simulink model "ofdm_txrx_alamouti"  (Simulink 6.3)
settings: Solver=ode45  StartTime=0.0  StopTime=45000  RelTol=1e-3
InitFcn:
  | ofdm_rx_alamouti_init
StartFcn:
  | ofdm_rx_alamouti_init
values from neighbouring files (symbols this model uses):
  CPLength = 16    [ofdm_tx_alamouti_init.m]
  CRCPolynomial = hex2dec('04c11db7')    [calcTxCRC.m]    (also in ofdm_tx_alamouti_init.m)
  hi = zeros(1,length(cci))    [ofdm_rx_alamouti_init.m]
  hr = zeros(1,length(ccr))    [ofdm_rx_alamouti_init.m]
  max_CP_length = 16    [ofdm_tx_alamouti_init.m]
  max_num_baseRateSymbols = 31    [ofdm_tx_alamouti_init.m]
  max_num_subcarriers = 64    [ofdm_tx_alamouti_init.m]
  max_num_trainingSymbols = 15    [ofdm_tx_alamouti_init.m]
  max_OFDM_symbols = 2047    [ofdm_tx_alamouti_init.m]
  modConstellation_qam16 = 0.75*[1 3 -1 -3]./3    [ofdm_tx_alamouti_init.m]
  modConstellation_qam256 = 0.9375*[3 1 5 7 11 9 13 15 -3 -1 -5 -7 -11 -9 -13 -15]./15    [ofdm_tx_alamouti_init.m]
  modConstellation_qam64 = 0.875*[3 1 5 7 -3 -1 -5 -7]./7    [ofdm_tx_alamouti_init.m]
  modConstellation_qpsk = [1 -1]./sqrt(2)    [ofdm_tx_alamouti_init.m]
  numBaseRateSymbols = 2    [ofdm_tx_alamouti_init.m]
  numFullRateSymbols = numFullRateSymbols + mod(numFullRateSymbols, 2)    [ofdm_tx_alamouti_init.m]
  numSubcarriers = 64    [ofdm_tx_alamouti_init.m]
  numTrainingSymbols = 4    [ofdm_tx_alamouti_init.m]
  pkt_crossCorr_thresh = 0.7    [ofdm_rx_alamouti_init.m]
  pkt_energy_thresh = 0    [ofdm_rx_alamouti_init.m]
  pkt_numPayloadBytes = 24+4+20+20+500    (= 568)    [ofdm_tx_alamouti_init.m]
  pktTiming_count_payloadStart = pktTiming_count_lsStart + pktTiming_ls_num*pktTiming_ls_length    [ofdm_rx_init_packetTimingControl.m]
  preamble = 6*[0 shortsyms_10 longsyms_2]    [ofdm_tx_alamouti_init.m]
  preamble_I = real(preamble)    [ofdm_tx_alamouti_init.m]
  preamble_Q = imag(preamble)    [ofdm_tx_alamouti_init.m]
  RAM_init_size = 4096    [ofdm_tx_alamouti_init.m]
  rx_longCorrCountLoad = 251-16    (= 235)    [ofdm_rx_alamouti_init.m]
  rx_longCorrThresh = 3000    [ofdm_rx_alamouti_init.m]
  RxDataScrambling_Seq = [40 198 78 63 82 173 102 245 48 111 172 115 147 230 216 93 72 65 62 2 205 242 122 90 128 83 105 97 73 10 5 252]    [ofdm_tx_alamouti_init.m]
  simOnly_numSamples = length(preamble)+( (numSubcarriers+CPLength)*(numTrainingSymbols + numBaseRateSymbols + numFullRateSymbols) )    [ofdm_tx_alamouti_init.m]
  train = [train train]    [ofdm_tx_alamouti_init.m]
  TxDataScrambling_Seq = [40 198 78 63 82 173 102 245 48 111 172 115 147 230 216 93 72 65 62 2 205 242 122 90 128 83 105 97 73 10 5 252]    [ofdm_tx_alamouti_init.m]
undefined symbols (not defined in the file or in its folder): CRC_table_gen, gcbh, interpFilter_4, xlBlockLoadCallback
libraries: xbsIndex_r4 x4373, dspmlti4 x4, dspsigops x4, commchan3 x2, simulink x2, commrflib2 x2, commsink2 x2, dspsrcs4 x1
(part 1 of 39)
settings Model: LastModifiedBy=murphpo

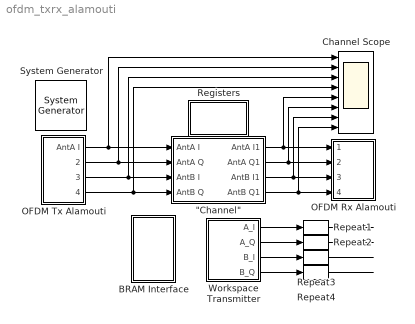
== system ofdm_txrx_alamouti ==
  " System Generator" : xbsIndex_r4/ System Generator  Tag=genX  xilinxfamily=Virtex2P  part=xc2vp70  speed=-6  package=ff1517  synthesis_tool=XST  directory=./opb_TxRx_Alamouti_2Rx_03  testbench=off  simulink_period=1  sysclk_period=20  incr_netlist=off  trim_vbits=Everywhere in SubSystem  dbl_ovrd=According to Block Masks  core_generation=According to Block Masks  run_coregen=off  deprecated_control=off  eval_field=0  block_type=sysgen
      settings: block_version=8.2.02
  "\"Channel\"" : Subsystem  mask{h=[1 0; 0 0], freqOffset=4e-4, awgnSNR=500, phNoise=-5000}
      mask prompts: h "2x2 Channel Matrix"; freqOffset "Freq Offset (phase inc [0,1])"; awgnSNR "SNR (dB)"; phNoise "Phase Noise"
      mask help: This block implements a simple static 2x2 channel. The one parameter sets the 2x2 channel matrix. h=[1 0;0 1] would be perfectly orthogonal channels. The norm of the matrix should be 1 to avoid overflow.
  BRAM Interface : Subsystem
  Channel Scope : Scope  NumInputPorts=8
      settings: TimeRange=10000  YMin=-1~-1~-1~-1~-3e-025~-3e-025~-3e-025~-3e-025  YMax=1~1~1~1~3e-025~3e-025~3e-025~3e-025  SaveName=ScopeData49
  OFDM Rx Alamouti : Subsystem
  OFDM Tx Alamouti : Subsystem
  Registers : Subsystem
  Repeat{1..4} (4 alike) : dspsigops/Repeat  N=4  ic=0  mode=Maintain input frame size
  Workspace Transmitter : Subsystem
  wires:
  OFDM Tx Alamouti/1(" AntA I") -> Channel Scope/1, "\"Channel\""/1(AntA I)
  OFDM Tx Alamouti/2(" AntA Q") -> "\"Channel\""/2(AntA Q), Channel Scope/2
  OFDM Tx Alamouti/3(" AntB I") -> "\"Channel\""/3(AntB I), Channel Scope/3
  OFDM Tx Alamouti/4(" AntB Q") -> "\"Channel\""/4(AntB Q), Channel Scope/4
  "\"Channel\""/1(AntA I1) -> Channel Scope/5, OFDM Rx Alamouti/1(AntA_I)
  "\"Channel\""/2(AntA Q1) -> Channel Scope/6, OFDM Rx Alamouti/2(AntA_Q)
  "\"Channel\""/3(AntB I1) -> Channel Scope/7, OFDM Rx Alamouti/3(AntB_I)
  "\"Channel\""/4(AntB Q1) -> Channel Scope/8, OFDM Rx Alamouti/4(AntB_Q)
  Workspace Transmitter/1(A_I) -> Repeat1/1
  Workspace Transmitter/2(A_Q) -> Repeat2/1
  Workspace Transmitter/3(B_I) -> Repeat3/1
  Workspace Transmitter/4(B_Q) -> Repeat4/1
  Repeat1/1 -> ?
  Repeat2/1 -> ?
  Repeat3/1 -> ?
  Repeat4/1 -> ?

== system ofdm_txrx_alamouti/"Channel" ==
  AntA I : Inport
  AntA Q : Inport  Port=2
  AntB I : Inport  Port=3
  AntB Q : Inport  Port=4
  AWGN Channel : commchan3/AWGN Channel  seed=rand  noiseMode="Signal to noise ratio  (SNR)"  EbNodB=10  EsNodB=10  SNRdB=awgnSNR  bitsPerSym=1  Ps=1  Tsym=1  variance=1
  AWGN Channel1 : commchan3/AWGN Channel  seed=rand  noiseMode="Signal to noise ratio  (SNR)"  EbNodB=10  EsNodB=10  SNRdB=awgnSNR  bitsPerSym=1  Ps=1  Tsym=1  variance=1
  Channel Scope : Scope  NumInputPorts=6
      settings: TimeRange=3000  YMin=-1~-1~-0.3~-0.3~-1~-1  YMax=0.75~0.75~0.2~0.2~1~1  SaveName=ScopeData52
  Complex to Real-Imag{1..5} (5 alike) : ComplexToRealImag
  FIR Decimation1 : dspmlti4/FIR Decimation  DialogController=dspDDGCreate  DialogControllerArgs=DataTag1  h=fir1(31, 1/4)  D=4  framing=Maintain input frame size  outputBufInitCond=0  roundingMode=Floor  overflowMode=off
  FIR Decimation2 : dspmlti4/FIR Decimation  DialogController=dspDDGCreate  DialogControllerArgs=DataTag2  h=fir1(31, 1/4)  D=4  framing=Maintain input frame size  outputBufInitCond=0  roundingMode=Floor  overflowMode=off
  FIR Decimation3 : dspmlti4/FIR Decimation  DialogController=dspDDGCreate  DialogControllerArgs=DataTag3  h=fir1(31, 1/4)  D=4  framing=Maintain input frame size  outputBufInitCond=0  roundingMode=Floor  overflowMode=off
  FIR Decimation4 : dspmlti4/FIR Decimation  DialogController=dspDDGCreate  DialogControllerArgs=DataTag4  h=fir1(31, 1/4)  D=4  framing=Maintain input frame size  outputBufInitCond=0  roundingMode=Floor  overflowMode=off
  Freq Offset : Subsystem
  Gain : Gain
  Gain1 : Gain
  Integer Delay : simulink/Discrete/Integer Delay  vinit=0.0  samptime=-1  NumDelays=1
  Integer Delay1 : simulink/Discrete/Integer Delay  vinit=0.0  samptime=-1  NumDelays=1
  "MIMO \"Channel\"" : Subsystem
  Phase Noise : commrflib2/Phase Noise  PhNs=phNoise  Fo=100  seed=rand
      settings: AttributesFormatString=\n
  Phase Noise1 : commrflib2/Phase Noise  PhNs=phNoise  Fo=100  seed=rand
      settings: AttributesFormatString=\n
  Real-Imag to Complex : RealImagToComplex
  Real-Imag to Complex1 : RealImagToComplex
  AntA I1 : Outport
  AntA Q1 : Outport  Port=2
  AntB I1 : Outport  Port=3
  AntB Q1 : Outport  Port=4
  wires:
  AntA I/1 -> Real-Imag to Complex/1
  AntA Q/1 -> Real-Imag to Complex/2
  AntB I/1 -> Real-Imag to Complex1/1
  AntB Q/1 -> Real-Imag to Complex1/2
  Complex to Real-Imag1/1 -> FIR Decimation3/1, AntA I1/1
  Complex to Real-Imag1/2 -> FIR Decimation4/1, AntA Q1/1
  "MIMO \"Channel\""/1(RxA) -> Freq Offset/1(In1), Complex to Real-Imag3/1
  Freq Offset/1(Out1) -> Gain/1, Complex to Real-Imag4/1
  "MIMO \"Channel\""/2(RxB) -> Freq Offset/2(In2)
  Gain/1 -> Integer Delay/1
  AWGN Channel/1 -> Complex to Real-Imag1/1
  Real-Imag to Complex/1 -> "MIMO \"Channel\""/1(TxA), Complex to Real-Imag2/1
  Real-Imag to Complex1/1 -> "MIMO \"Channel\""/2(TxB)
  Phase Noise/1 -> AWGN Channel/1
  FIR Decimation3/1 -> ?
  FIR Decimation4/1 -> ?
  Integer Delay/1 -> Phase Noise/1
  Complex to Real-Imag2/1 -> Channel Scope/1
  Complex to Real-Imag2/2 -> Channel Scope/2
  Complex to Real-Imag3/1 -> Channel Scope/3
  Complex to Real-Imag3/2 -> Channel Scope/4
  Complex to Real-Imag4/1 -> Channel Scope/5
  Complex to Real-Imag4/2 -> Channel Scope/6
  Complex to Real-Imag5/1 -> FIR Decimation1/1, AntB I1/1
  Complex to Real-Imag5/2 -> FIR Decimation2/1, AntB Q1/1
  Gain1/1 -> Integer Delay1/1
  AWGN Channel1/1 -> Complex to Real-Imag5/1
  Phase Noise1/1 -> AWGN Channel1/1
  FIR Decimation1/1 -> ?
  FIR Decimation2/1 -> ?
  Integer Delay1/1 -> Phase Noise1/1
  Freq Offset/2(Out2) -> Gain1/1

== system ofdm_txrx_alamouti/BRAM Interface ==
  " 19b1" : xbsIndex_r4/Constant  arith_type=Unsigned  const=0  n_bits=32-2-9-3  bin_pt=0  explicit_period=on  period=1  equ=P=C  opselect=C  inp2=PCIN>>17  opr=+  inp1=P  carry=CIN  dbl_ovrd=off  block_type=constant
      settings: block_version=8.2.02
  " 3b1" : xbsIndex_r4/Constant  arith_type=Unsigned  const=0  n_bits=3  bin_pt=0  explicit_period=on  period=1  equ=P=C  opselect=C  inp2=PCIN>>17  opr=+  inp1=P  carry=CIN  dbl_ovrd=off  block_type=constant
      settings: block_version=8.2.02
  19b1 : xbsIndex_r4/Constant  arith_type=Unsigned  const=0  n_bits=32-2-9-3  bin_pt=0  explicit_period=on  period=1  equ=P=C  opselect=C  inp2=PCIN>>17  opr=+  inp1=P  carry=CIN  dbl_ovrd=off  block_type=constant
      settings: block_version=8.2.02
  2LSB : xbsIndex_r4/Slice  nbits=2  boolean_output=off  mode=Lower Bit Location + Width  bit1=0  base1=MSB of Input  bit0=0  base0=LSB of Input  dbl_ovrd=off  block_type=slice
      settings: infoedit="Extracts a given range of bits from each input sample and presents it at the output.  The output type is ordinarily unsigned with binary point at zero, but can be Boolean when the slice is one bit wide.<P><P>Hardware notes: In hardware this block costs nothing."  block_version=VER_STRING_GOES_HERE
  2MSB : xbsIndex_r4/Slice  nbits=2  boolean_output=off  mode=Upper Bit Location + Width  bit1=0  base1=MSB of Input  bit0=0  base0=LSB of Input  dbl_ovrd=off  block_type=slice
      settings: infoedit="Extracts a given range of bits from each input sample and presents it at the output.  The output type is ordinarily unsigned with binary point at zero, but can be Boolean when the slice is one bit wide.<P><P>Hardware notes: In hardware this block costs nothing."  block_version=VER_STRING_GOES_HERE
  3b1 : xbsIndex_r4/Constant  arith_type=Unsigned  const=0  n_bits=3  bin_pt=0  explicit_period=on  period=1  equ=P=C  opselect=C  inp2=PCIN>>17  opr=+  inp1=P  carry=CIN  dbl_ovrd=off  block_type=constant
      settings: block_version=8.2.02
  BRAM_Addr : xbsIndex_r4/Gateway Out  hdl_port=on  timing_constraint=None  locs_specified=off  LOCs={}  block_type=gatewayout
      settings: infoedit="Gateway out block.  Converts Xilinx fixed point inputs into ouputs of type Simulink integer, double, or fixed point.<P><P>Hardware notes:  In hardware these blocks become top level output ports or are discarded, depending on how they are configured."  xl_area=[0, 0, 0, 0, 0, 0, 0]  block_version=VER_STRING_GOES_HERE
  BRAM_DataIn : xbsIndex_r4/Gateway In  arith_type=Unsigned  n_bits=64  bin_pt=0  quantization="Round  (unbiased: +/- Inf)"  overflow=Saturate  period=1  dbl_ovrd=off  timing_constraint=None  locs_specified=off  LOCs={}  block_type=gatewayin
      settings: infoedit="Gateway in block.  Converts inputs of type Simulink integer, double and fixed point to  Xilinx fixed point type.<P><P>Hardware notes:  In hardware these blocks become top level input ports."  block_version=VER_STRING_GOES_HERE
  BRAM_Dout : xbsIndex_r4/Gateway Out  hdl_port=on  timing_constraint=None  locs_specified=off  LOCs={}  block_type=gatewayout
      settings: infoedit="Gateway out block.  Converts Xilinx fixed point inputs into ouputs of type Simulink integer, double, or fixed point.<P><P>Hardware notes:  In hardware these blocks become top level output ports or are discarded, depending on how they are configured."  xl_area=[0, 0, 0, 0, 0, 0, 0]  block_version=VER_STRING_GOES_HERE
  BRAM_Reset : xbsIndex_r4/Gateway Out  hdl_port=on  timing_constraint=None  locs_specified=off  LOCs={}  block_type=gatewayout
      settings: infoedit="Gateway out block.  Converts Xilinx fixed point inputs into ouputs of type Simulink integer, double, or fixed point.<P><P>Hardware notes:  In hardware these blocks become top level output ports or are discarded, depending on how they are configured."  block_version=8.2.02
  BRAM_WEn : xbsIndex_r4/Gateway Out  hdl_port=on  timing_constraint=None  locs_specified=off  LOCs={}  block_type=gatewayout
      settings: infoedit="Gateway out block.  Converts Xilinx fixed point inputs into ouputs of type Simulink integer, double, or fixed point.<P><P>Hardware notes:  In hardware these blocks become top level output ports or are discarded, depending on how they are configured."  xl_area=[0, 0, 0, 0, 0, 0, 0]  block_version=VER_STRING_GOES_HERE
  Concat3 : xbsIndex_r4/Concat  num_inputs=4  dbl_ovrd=off  block_type=concat
      settings: block_version=8.2.02
  Concat4 : xbsIndex_r4/Concat  num_inputs=4  dbl_ovrd=off  block_type=concat
      settings: block_version=8.2.02
  Constant : xbsIndex_r4/Constant  arith_type=Unsigned  const=0  n_bits=1  bin_pt=0  explicit_period=on  period=1  equ=P=C  opselect=C  inp2=PCIN>>17  opr=+  inp1=P  carry=CIN  dbl_ovrd=off  block_type=constant
      settings: block_version=8.2.02
  Mux : xbsIndex_r4/Mux  inputs=2  en=off  latency=0  precision=Full  arith_type=Unsigned  n_bits=16  bin_pt=14  quantization=Truncate  overflow=Wrap  dbl_ovrd=off  block_type=mux
      settings: block_version=8.2.02
  PLB Pkt Buffer : Subsystem
  tag scope: BRAM_Tx_DIn=global, Debug_BRAM_Addr=global
  wires:
  PLB Pkt Buffer/1(Data) -> BRAM_DataIn/1
  [BRAM_Rx_DOut] -> BRAM_Dout/1
  [BRAM_Rx_WrEn] -> BRAM_WEn/1
  BRAM_DataIn/1 -> [BRAM_Tx_DIn]
  Constant/1 -> BRAM_Reset/1
  Mux/1 -> BRAM_Addr/1, [Debug_BRAM_Addr]
  [BRAM_Rx_AnyWrEn] -> Mux/1
  BRAM_Dout/1 -> PLB Pkt Buffer/1(BRAM_Dout)
  BRAM_WEn/1 -> PLB Pkt Buffer/2(WEn)
  BRAM_Addr/1 -> PLB Pkt Buffer/3(Addr)
  Concat4/1 -> Mux/2
  Concat3/1 -> Mux/3
  [BRAM_Rx_WrAddr] -> Concat3/3
  2MSB/1 -> Concat3/2
  19b1/1 -> Concat3/1
  " 3b1"/1 -> Concat4/4
  [BRAM_Tx_RdAddr] -> Concat4/3
  2LSB/1 -> Concat4/2
  " 19b1"/1 -> Concat4/1
  [regTxRx_PktBuffOffsets] -> 2LSB/1, 2MSB/1
  3b1/1 -> Concat3/4

== system ofdm_txrx_alamouti/OFDM Rx Alamouti ==
  AntA_I : Inport
  AntA_Q : Inport  Port=2
  AntB_I : Inport  Port=3
  AntB_Q : Inport  Port=4
  ADCs & Ant Selection : Subsystem
  ChipScope : xbsIndex_r4/ChipScope  num_trig_ports=5  current_port=4  show_trig_port=0  match_units=1  match_type=Basic with edges  data_is_trigger=off  num_data_ports=12  data_depth=8192  SRL16s=on  match_type_t0=2  match_type_t1=2  match_type_t2=2  match_type_t3=2  match_type_t4=2  match_type_t5=1  match_type_t6=1  match_type_t7=1  match_type_t8=1  match_type_t9=1  match_type_t10=1  match_type_t11=1  match_type_t12=1  match_type_t13=1  match_type_t14=1  match_type_t15=1  match_units_t0=1  match_units_t1=1  match_units_t2=1  match_units_t3=1  match_units_t4=1  match_units_t5=1  match_units_t6=1  match_units_t7=1  match_units_t8=1  match_units_t9=1  match_units_t10=1  match_units_t11=1  match_units_t12=1  match_units_t13=1  match_units_t14=1  match_units_t15=1  dbl_ovrd=0  block_type=chipscope
      settings: infoedit="Enables run-time debugging and verification of signals by inserting ChipScope Pro ICON and ILA cores.<br><br>  Restrictions: <br>Only one ChipScope core can be instantiated in a System Generator design.This block cannot be used at the same time as JTAG hardware co-simulation.A design or subsystem containing a ChipScope block must have at least one output port."  block_version=VER_STRING_GOES_HERE
  Coarse Freq Correction1 : Subsystem
  Constant : Constant  Value=0
  Counter1 : xbsIndex_r4/Counter  cnt_type=Free Running  cnt_to=Inf  operation=Up  start_count=0  cnt_by_val=1  arith_type=Unsigned  n_bits=2  bin_pt=0  load_pin=off  rst=off  en=off  explicit_period=on  period=1  dbl_ovrd=off  use_behavioral_HDL=off  use_rpm=on  block_type=counter
      settings: xl_area=[5 10 0 10 0 0 0]  block_version=VER_STRING_GOES_HERE
  Display : Display
  Display1 : Display
  Display2 : Display
  Divide : Product  Inputs=*/  OutScaling=2^-10
  Equalizer & Packetizer : Subsystem
  FFT & Chan Est : Subsystem
  Long Corr 4x : Subsystem
  Packet_Detection : Subsystem
  Packet_Timing_Control : Subsystem
  Rx Input : Scope  NumInputPorts=5
      settings: TimeRange=3500  YMin=-0.25~-0.25~0~0~0  YMax=0.25~0.25~1~12500~1  SaveName=ScopeData48
  Slice2 : xbsIndex_r4/Slice  nbits=1  boolean_output=off  mode=Upper Bit Location + Width  bit1=0  base1=MSB of Input  bit0=0  base0=LSB of Input  dbl_ovrd=off  block_type=slice
      settings: infoedit="Extracts a given range of bits from each input sample and presents it at the output.  The output type is ordinarily unsigned with binary point at zero, but can be Boolean when the slice is one bit wide.<P><P>Hardware notes: In hardware this block costs nothing."  block_version=VER_STRING_GOES_HERE
  Up Sample{,2,5,7,12} (5 alike) : xbsIndex_r4/Up Sample  sample_ratio=4  copy_samples=on  dbl_ovrd=off  block_type=usamp
      settings: infoedit="Up samples input data.  Inserted values  can be zeros or copies of the most recent input sample.<P><P>Hardware notes: No hardware is needed if inserted values are copies of the input sample; otherwise, a mux and single bit flip-flop are used."  block_version=VER_STRING_GOES_HERE
  Up Sample{1,3..4,6,9..11} (7 alike) : xbsIndex_r4/Up Sample  sample_ratio=2  copy_samples=on  dbl_ovrd=off  block_type=usamp
      settings: infoedit="Up samples input data.  Inserted values  can be zeros or copies of the most recent input sample.<P><P>Hardware notes: No hardware is needed if inserted values are copies of the input sample; otherwise, a mux and single bit flip-flop are used."  block_version=VER_STRING_GOES_HERE
  debug_chipscopeTrig : xbsIndex_r4/Gateway In  arith_type=Boolean  n_bits=14  bin_pt=13  quantization="Round  (unbiased: +/- Inf)"  overflow=Saturate  period=1  dbl_ovrd=off  timing_constraint=None  locs_specified=off  LOCs={}  block_type=gatewayin
      settings: infoedit="Gateway in block.  Converts inputs of type Simulink integer, double and fixed point to  Xilinx fixed point type.<P><P>Hardware notes:  In hardware these blocks become top level input ports."  block_version=VER_STRING_GOES_HERE
  done{1..2,4..5,13} (5 alike) : xbsIndex_r4/Gateway Out  hdl_port=off  timing_constraint=None  locs_specified=off  LOCs={}  block_type=gatewayout
      settings: infoedit="Gateway out block.  Converts Xilinx fixed point inputs into ouputs of type Simulink integer, double, or fixed point.<P><P>Hardware notes:  In hardware these blocks become top level output ports or are discarded, depending on how they are configured."  xl_area=[0 0 0 0 1 0 0]  block_version=VER_STRING_GOES_HERE
  tag scope: Payload=global
  wires:
  Packet_Detection/1(Pkt Det) -> done1/1, Packet_Timing_Control/1(Pkt Det)
  Packet_Timing_Control/1(Payload_Preamble) -> Equalizer & Packetizer/1(Payload), FFT & Chan Est/1(Payload), [Payload], done13/1
  Long Corr 4x/1(y) -> Packet_Timing_Control/2(Long Corr), done2/1
  done1/1 -> Rx Input/3
  done4/1 -> Rx Input/1
  done5/1 -> Rx Input/2
  done2/1 -> Rx Input/4
  Equalizer & Packetizer/1(Fine Freq) -> Coarse Freq Correction1/5(Fine Freq)
  Equalizer & Packetizer/2(done) -> Packet_Timing_Control/3(Done), Coarse Freq Correction1/6(PktDone)
  [Payload] -> Up Sample/1
  [AntA_ADC_I_Filt] -> Up Sample7/1
  [EQ_I] -> Up Sample3/1
  [EQ_Q] -> Up Sample4/1
  [Long_Corr] -> Up Sample2/1
  [FFT_I] -> Up Sample6/1
  Constant/1 -> debug_chipscopeTrig/1
  [ChanEst_I_EQ] -> Up Sample9/1
  Up Sample/1 -> ChipScope/6, ChipScope/1  "Payload"
  Up Sample2/1 -> ChipScope/13  "LongCorr"
  Up Sample3/1 -> ChipScope/11  "EQ_I"
  Up Sample4/1 -> ChipScope/12  "EQ_Q"
  Up Sample6/1 -> ChipScope/14  "FFT_I"
  Up Sample7/1 -> ChipScope/9  "AntA_ADC_I"
  debug_chipscopeTrig/1 -> ChipScope/2  "ExtTrig"
  Up Sample9/1 -> ChipScope/15  "ChanEstA I"
  Up Sample10/1 -> ChipScope/16  "PilotPhaseErr"
  FFT & Chan Est/1(Y_I) -> Equalizer & Packetizer/2(Y_I)
  FFT & Chan Est/2(Y_Q) -> Equalizer & Packetizer/3(Y_Q)
  FFT & Chan Est/3(H-I) -> Equalizer & Packetizer/4(H-I)
  FFT & Chan Est/4(H-Q) -> Equalizer & Packetizer/5(H-Q)
  FFT & Chan Est/5(SubCar Index) -> Equalizer & Packetizer/6(SubCar Index)
  FFT & Chan Est/6(Sym Index) -> Equalizer & Packetizer/7(Sym Index)
  FFT & Chan Est/7(Vout) -> Equalizer & Packetizer/8(Vin)
  Up Sample5/1 -> ChipScope/17  "CFO Phase Inc"
  [CFO_PhaseInc] -> Up Sample5/1
  Packet_Timing_Control/2(PktReset) -> ADCs & Ant Selection/5(PktDetReset), Coarse Freq Correction1/2(Reset)
  done13/1 -> Rx Input/5
  Coarse Freq Correction1/1(" AntA I") -> FFT & Chan Est/2(AntA I), Long Corr 4x/1(I In)
  Coarse Freq Correction1/2(" AntA Q") -> FFT & Chan Est/3(AntA Q), Long Corr 4x/2(Q In)
  [GoodPktInt] -> Up Sample1/1
  [BadPktInt] -> Up Sample11/1
  Up Sample1/1 -> ChipScope/7, ChipScope/3  "Good Pkt Int"
  Up Sample11/1 -> ChipScope/8, ChipScope/4  "Bad Pkt Int"
  [AntA_ADC_Q_Filt] -> Up Sample12/1
  Up Sample12/1 -> ChipScope/10  "AntA_ADC_Q"
  AntA_I/1 -> ADCs & Ant Selection/1("A I ")
  AntA_Q/1 -> ADCs & Ant Selection/2("A Q ")
  ADCs & Ant Selection/1(I) -> Packet_Detection/1(Ant I), done4/1, Coarse Freq Correction1/3(AntA I)
  ADCs & Ant Selection/2(Q) -> Packet_Detection/2(Ant Q), done5/1, Coarse Freq Correction1/4(AntA Q)
  AntB_I/1 -> ADCs & Ant Selection/3("B I ")
  AntB_Q/1 -> ADCs & Ant Selection/4("B Q ")
  [PilotPhaseErr] -> Up Sample10/1
  Counter1/1 -> Slice2/1
  Slice2/1 -> ChipScope/5  "SampClk"
  Divide/1 -> Display/1
  [BER_Errors] -> Divide/1, Display1/1
  [BER_TotalBits] -> Divide/2, Display2/1
  Packet_Timing_Control/3(PktDetected) -> Coarse Freq Correction1/1(PktDet)

== system ofdm_txrx_alamouti/OFDM Tx Alamouti ==
  Filter & DACs : Subsystem
  IFFT : Subsystem
  OutputBuffers : Subsystem
  OutputMuxes : Subsystem
  PreCFO : Subsystem
  Restarter : Subsystem
  Training_Data : Subsystem  Orientation=left
  Tx Scope : Scope  NumInputPorts=8
      settings: TimeRange=3000  YMin=-1~-1~-1~-0.0932617~0.0311279~-0.0801392~-1~-1  YMax=1~1~1~-0.0932617~0.0311279~-0.0801392~1~1  SaveName=ScopeData30
  TxControl : Subsystem
  TxStart : Subsystem
  done{1..8} (8 alike) : xbsIndex_r4/Gateway Out  hdl_port=off  timing_constraint=None  locs_specified=off  LOCs={}  block_type=gatewayout
      settings: infoedit="Gateway out block.  Converts Xilinx fixed point inputs into ouputs of type Simulink integer, double, or fixed point.<P><P>Hardware notes:  In hardware these blocks become top level output ports or are discarded, depending on how they are configured."  xl_area=[0 0 0 0 1 0 0]  block_version=VER_STRING_GOES_HERE
  " AntA I" : Outport
  " AntA Q" : Outport  Port=2
  " AntB I" : Outport  Port=3
  " AntB Q" : Outport  Port=4
  wires:
  Restarter/1(Start) -> TxControl/1(StartTx), Training_Data/5(start), done4/1
  Training_Data/1(tx_Q) -> IFFT/2(xn_Q), done8/1
  TxControl/6(Start IFFT) -> IFFT/3(Start), done2/1
  IFFT/3(xk_I) -> OutputBuffers/2(I), done6/1
  IFFT/4(xk_Q) -> OutputBuffers/3(Q)
  IFFT/1(xn_Index) -> Training_Data/7(xn_index)
  IFFT/5(xk_Index) -> OutputBuffers/4(Xk_index)
  OutputBuffers/1(AntA_I) -> OutputMuxes/2(AntA I), done3/1
  OutputBuffers/2(AntA_Q) -> OutputMuxes/3(AntA Q), done5/1
  TxControl/4(Training_Data) -> Training_Data/2(train_pay)
  TxControl/5(CPn_Data) -> Training_Data/1(CPn_Data)
  TxControl/3(BaseRate_En) -> Training_Data/3(BaseRate_En)
  OutputBuffers/5(Vout) -> OutputMuxes/6(vin)
  Training_Data/2(tx_I) -> IFFT/1(xn_I), done7/1
  OutputBuffers/6(Pkt Done) -> OutputMuxes/7(Pkt Done), Training_Data/6(packet_done), TxControl/2(PktDone), Restarter/2(Done), done1/1
  OutputBuffers/3(AntB_I) -> OutputMuxes/4(AntB I)
  OutputBuffers/4(AntB_Q) -> OutputMuxes/5(AntB Q)
  TxControl/1(Preamble Index) -> OutputMuxes/1(Pream Index)
  IFFT/2(Vout) -> OutputBuffers/1(IFFT Vout)
  TxControl/2(FFT En) -> Training_Data/4(FFT En)
  OutputMuxes/1(" AntA I") -> PreCFO/1(" AI")
  OutputMuxes/2(" AntA Q") -> PreCFO/2(" AQ")
  OutputMuxes/3(" AntB I") -> PreCFO/3(" BI")
  OutputMuxes/4(" AntB Q") -> PreCFO/4(" BQ")
  TxStart/1(Start) -> Restarter/1(StartTx)
  Restarter/2(2ndTx) -> OutputMuxes/8(2ndTx)
  done4/1 -> Tx Scope/1
  done1/1 -> Tx Scope/2
  done2/1 -> Tx Scope/3
  done3/1 -> Tx Scope/4
  done5/1 -> Tx Scope/5
  done6/1 -> Tx Scope/6
  done7/1 -> Tx Scope/7
  done8/1 -> Tx Scope/8
  PreCFO/1(AI) -> Filter & DACs/1(" AI")
  PreCFO/2(AQ) -> Filter & DACs/2(" AQ")
  PreCFO/3(BI) -> Filter & DACs/3(" BI")
  PreCFO/4(BQ) -> Filter & DACs/4(" BQ")
  Filter & DACs/1(AI) -> " AntA I"/1
  Filter & DACs/2(AQ) -> " AntA Q"/1
  Filter & DACs/3(BI) -> " AntB I"/1
  Filter & DACs/4(BQ) -> " AntB Q"/1

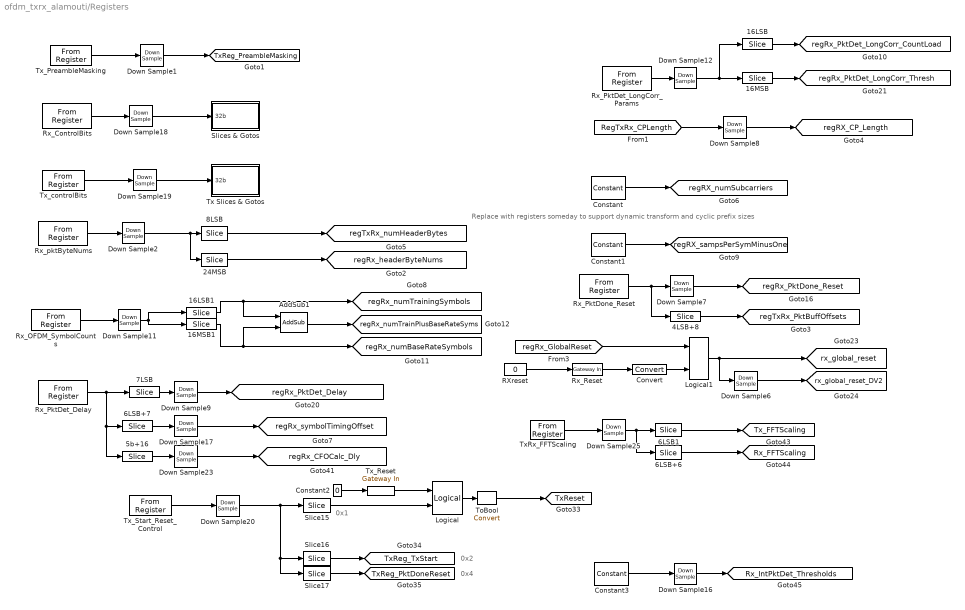
== system ofdm_txrx_alamouti/Registers ==
  note: Replace with registers someday
      | to support dynamic transform and
      | cyclic prefix sizes
  note: 0x2
  note: 0x1
  note: 0x4
  16LSB : xbsIndex_r4/Slice  nbits=16  boolean_output=off  mode=Lower Bit Location + Width  bit1=0  base1=MSB of Input  bit0=0  base0=LSB of Input  dbl_ovrd=off  block_type=slice
      settings: infoedit="Extracts a given range of bits from each input sample and presents it at the output.  The output type is ordinarily unsigned with binary point at zero, but can be Boolean when the slice is one bit wide.<P><P>Hardware notes: In hardware this block costs nothing."  block_version=VER_STRING_GOES_HERE
  16LSB1 : xbsIndex_r4/Slice  nbits=ceil(log2(max_num_trainingSymbols))  boolean_output=off  mode=Lower Bit Location + Width  bit1=0  base1=MSB of Input  bit0=0  base0=LSB of Input  dbl_ovrd=off  block_type=slice
      settings: infoedit="Extracts a given range of bits from each input sample and presents it at the output.  The output type is ordinarily unsigned with binary point at zero, but can be Boolean when the slice is one bit wide.<P><P>Hardware notes: In hardware this block costs nothing."  block_version=VER_STRING_GOES_HERE
  16MSB : xbsIndex_r4/Slice  nbits=16  boolean_output=off  mode=Lower Bit Location + Width  bit1=0  base1=MSB of Input  bit0=16  base0=LSB of Input  dbl_ovrd=off  block_type=slice
      settings: infoedit="Extracts a given range of bits from each input sample and presents it at the output.  The output type is ordinarily unsigned with binary point at zero, but can be Boolean when the slice is one bit wide.<P><P>Hardware notes: In hardware this block costs nothing."  block_version=VER_STRING_GOES_HERE
  16MSB1 : xbsIndex_r4/Slice  nbits=ceil(log2(max_num_baseRateSymbols))  boolean_output=off  mode=Lower Bit Location + Width  bit1=0  base1=MSB of Input  bit0=16  base0=LSB of Input  dbl_ovrd=off  block_type=slice
      settings: infoedit="Extracts a given range of bits from each input sample and presents it at the output.  The output type is ordinarily unsigned with binary point at zero, but can be Boolean when the slice is one bit wide.<P><P>Hardware notes: In hardware this block costs nothing."  block_version=VER_STRING_GOES_HERE
  24MSB : xbsIndex_r4/Slice  nbits=24  boolean_output=off  mode=Lower Bit Location + Width  bit1=0  base1=MSB of Input  bit0=8  base0=LSB of Input  dbl_ovrd=off  block_type=slice
      settings: infoedit="Extracts a given range of bits from each input sample and presents it at the output.  The output type is ordinarily unsigned with binary point at zero, but can be Boolean when the slice is one bit wide.<P><P>Hardware notes: In hardware this block costs nothing."  block_version=VER_STRING_GOES_HERE
  4LSB+8 : xbsIndex_r4/Slice  nbits=4  boolean_output=off  mode=Lower Bit Location + Width  bit1=0  base1=MSB of Input  bit0=8  base0=LSB of Input  dbl_ovrd=off  block_type=slice
      settings: infoedit="Extracts a given range of bits from each input sample and presents it at the output.  The output type is ordinarily unsigned with binary point at zero, but can be Boolean when the slice is one bit wide.<P><P>Hardware notes: In hardware this block costs nothing."  block_version=VER_STRING_GOES_HERE
  5b+16 : xbsIndex_r4/Slice  nbits=5  boolean_output=off  mode=Lower Bit Location + Width  bit1=0  base1=MSB of Input  bit0=16  base0=LSB of Input  dbl_ovrd=off  block_type=slice
      settings: infoedit="Extracts a given range of bits from each input sample and presents it at the output.  The output type is ordinarily unsigned with binary point at zero, but can be Boolean when the slice is one bit wide.<P><P>Hardware notes: In hardware this block costs nothing."  block_version=VER_STRING_GOES_HERE
  6LSB+6 : xbsIndex_r4/Slice  nbits=6  boolean_output=off  mode=Lower Bit Location + Width  bit1=0  base1=MSB of Input  bit0=6  base0=LSB of Input  dbl_ovrd=off  block_type=slice
      settings: infoedit="Extracts a given range of bits from each input sample and presents it at the output.  The output type is ordinarily unsigned with binary point at zero, but can be Boolean when the slice is one bit wide.<P><P>Hardware notes: In hardware this block costs nothing."  block_version=VER_STRING_GOES_HERE
  6LSB+7 : xbsIndex_r4/Slice  nbits=6  boolean_output=off  mode=Lower Bit Location + Width  bit1=0  base1=MSB of Input  bit0=7  base0=LSB of Input  dbl_ovrd=off  block_type=slice
      settings: infoedit="Extracts a given range of bits from each input sample and presents it at the output.  The output type is ordinarily unsigned with binary point at zero, but can be Boolean when the slice is one bit wide.<P><P>Hardware notes: In hardware this block costs nothing."  block_version=VER_STRING_GOES_HERE
  6LSB1 : xbsIndex_r4/Slice  nbits=6  boolean_output=off  mode=Lower Bit Location + Width  bit1=0  base1=MSB of Input  bit0=0  base0=LSB of Input  dbl_ovrd=off  block_type=slice
      settings: infoedit="Extracts a given range of bits from each input sample and presents it at the output.  The output type is ordinarily unsigned with binary point at zero, but can be Boolean when the slice is one bit wide.<P><P>Hardware notes: In hardware this block costs nothing."  block_version=VER_STRING_GOES_HERE
  7LSB : xbsIndex_r4/Slice  nbits=7  boolean_output=off  mode=Lower Bit Location + Width  bit1=0  base1=MSB of Input  bit0=0  base0=LSB of Input  dbl_ovrd=off  block_type=slice
      settings: infoedit="Extracts a given range of bits from each input sample and presents it at the output.  The output type is ordinarily unsigned with binary point at zero, but can be Boolean when the slice is one bit wide.<P><P>Hardware notes: In hardware this block costs nothing."  block_version=VER_STRING_GOES_HERE
  "8LSB " : xbsIndex_r4/Slice  nbits=8  boolean_output=off  mode=Lower Bit Location + Width  bit1=0  base1=MSB of Input  bit0=0  base0=LSB of Input  dbl_ovrd=off  block_type=slice
      settings: infoedit="Extracts a given range of bits from each input sample and presents it at the output.  The output type is ordinarily unsigned with binary point at zero, but can be Boolean when the slice is one bit wide.<P><P>Hardware notes: In hardware this block costs nothing."  block_version=VER_STRING_GOES_HERE
  AddSub1 : xbsIndex_r4/AddSub  mode=Addition  use_carryin=off  use_carryout=off  en=off  latency=0  precision=User Defined  arith_type=Unsigned  n_bits=1 + ceil(log2(max_num_trainingSymbols + max_num_baseRateSymbols))  bin_pt=0  quantization=Truncate  overflow=Wrap  dbl_ovrd=off  use_behavioral_HDL=on  pipelined=off  use_rpm=on  block_type=addsub
      settings: xl_area=[0, 0, 0, 0, 0, 0, 0]  block_version=VER_STRING_GOES_HERE
  Constant : xbsIndex_r4/Constant  arith_type=Unsigned  const=numSubcarriers  n_bits=ceil(log2(max_num_subcarriers)+1)  bin_pt=0  explicit_period=on  period=2  equ=P=C  opselect=C  inp2=PCIN>>17  opr=+  inp1=P  carry=CIN  dbl_ovrd=off  block_type=constant
      settings: block_version=VER_STRING_GOES_HERE
  Constant1 : xbsIndex_r4/Constant  arith_type=Unsigned  const=numSubcarriers+CPLength-1  n_bits=ceil(log2(max_num_subcarriers+CPLength)+1)  bin_pt=0  explicit_period=on  period=2  equ=P=C  opselect=C  inp2=PCIN>>17  opr=+  inp1=P  carry=CIN  dbl_ovrd=off  block_type=constant
      settings: block_version=VER_STRING_GOES_HERE
  Constant2 : Constant  Value=0
  Constant3 : xbsIndex_r4/Constant  arith_type=Unsigned  const=(2^8 * pkt_energy_thresh) + pkt_crossCorr_thresh  n_bits=32  bin_pt=0  explicit_period=on  period=1  equ=P=C  opselect=C  inp2=PCIN>>17  opr=+  inp1=P  carry=CIN  dbl_ovrd=off  block_type=constant
      settings: block_version=VER_STRING_GOES_HERE
  Convert : xbsIndex_r4/Convert  arith_type=Boolean  n_bits=8  bin_pt=6  quantization=Truncate  overflow=Wrap  latency=0  dbl_ovrd=off  pipeline=off  block_type=convert
      settings: xl_area=[0, 0, 0, 0, 0, 0, 0]  block_version=VER_STRING_GOES_HERE
  Down Sample{1,9,12,16,23} (5 alike) : xbsIndex_r4/Down Sample  sample_ratio=4  sample_phase="Last Value of Frame  (most efficient)"  en=off  latency=1  dbl_ovrd=off  block_type=dsamp
      settings: block_version=VER_STRING_GOES_HERE
  Down Sample{2,6..8,11,17..20,25} (10 alike) : xbsIndex_r4/Down Sample  sample_ratio=2  sample_phase="Last Value of Frame  (most efficient)"  en=off  latency=1  dbl_ovrd=off  block_type=dsamp
      settings: block_version=VER_STRING_GOES_HERE
  Logical : xbsIndex_r4/Logical  logical_function=OR  inputs=2  en=off  latency=0  precision=Full  arith_type=Unsigned  n_bits=16  bin_pt=0  align_bp=on  dbl_ovrd=off  block_type=logical
      settings: block_version=VER_STRING_GOES_HERE
  Logical1 : xbsIndex_r4/Logical  logical_function=OR  inputs=2  en=off  latency=0  precision=Full  arith_type=Unsigned  n_bits=8  bin_pt=2  align_bp=on  dbl_ovrd=off  block_type=logical
      settings: xl_area=[1 0 0 1 0 0 0]  block_version=VER_STRING_GOES_HERE
  RXreset : Constant  Value=0
  Rx_ControlBits : xbsIndex_r4/From Register  shared_memory_name='RxReg_ControlBits'  init=rx_controlBits  period=1  ownership=Locally owned and initialized  arith_type=Unsigned  n_bits=32  bin_pt=0  dbl_ovrd=off  block_type=fromreg
      settings: AttributesFormatString=<< %<shared_memory_name> >>  infoedit="Register block that reads data to a shared memory register.  Delay of one sample period."  block_version=VER_STRING_GOES_HERE
  Rx_OFDM_SymbolCounts : xbsIndex_r4/From Register  shared_memory_name='Rx_OFDM_SymbolCounts'  init=(2^16 * numBaseRateSymbols) + numTrainingSymbols  period=1  ownership=Locally owned and initialized  arith_type=Unsigned  n_bits=32  bin_pt=0  dbl_ovrd=off  block_type=fromreg
      settings: AttributesFormatString=<< %<shared_memory_name> >>  infoedit="Register block that reads data to a shared memory register.  Delay of one sample period."  block_version=VER_STRING_GOES_HERE
  Rx_PktDet_Delay : xbsIndex_r4/From Register  shared_memory_name='Rx_PktDet_Delay'  init=PktDet_Delay  period=1  ownership=Locally owned and initialized  arith_type=Unsigned  n_bits=32  bin_pt=0  dbl_ovrd=off  block_type=fromreg
      settings: AttributesFormatString=<< %<shared_memory_name> >>  infoedit="Register block that reads data to a shared memory register.  Delay of one sample period."  block_version=VER_STRING_GOES_HERE
  Rx_PktDet_LongCorr_Params : xbsIndex_r4/From Register  shared_memory_name='Rx_PktDet_LongCorr_Params'  init=rx_longCorrCountLoad + (2^16 * rx_longCorrThresh)  period=1  ownership=Locally owned and initialized  arith_type=Unsigned  n_bits=32  bin_pt=0  dbl_ovrd=off  block_type=fromreg
      settings: AttributesFormatString=<< %<shared_memory_name> >>  infoedit="Register block that reads data to a shared memory register.  Delay of one sample period."  block_version=VER_STRING_GOES_HERE
  Rx_PktDone_Reset : xbsIndex_r4/From Register  shared_memory_name='Rx_PktDone_Reset'  init=hex2dec('00') + (1 + 0)*2^8  period=1  ownership=Locally owned and initialized  arith_type=Unsigned  n_bits=32  bin_pt=0  dbl_ovrd=off  block_type=fromreg
      settings: AttributesFormatString=<< %<shared_memory_name> >>  infoedit="Register block that reads data to a shared memory register.  Delay of one sample period."  block_version=VER_STRING_GOES_HERE
  Rx_Reset : xbsIndex_r4/Gateway In  arith_type=Boolean  n_bits=6  bin_pt=0  quantization="Round  (unbiased: +/- Inf)"  overflow=Saturate  period=4  dbl_ovrd=off  timing_constraint=None  locs_specified=off  LOCs={}  block_type=gatewayin
      settings: infoedit="Gateway in block.  Converts inputs of type Simulink integer, double and fixed point to  Xilinx fixed point type.<P><P>Hardware notes:  In hardware these blocks become top level input ports."  xl_area=[0 0 0 0 1 0 0]  block_version=VER_STRING_GOES_HERE
  Rx_pktByteNums : xbsIndex_r4/From Register  shared_memory_name='Rx_pktByteNums'  init=pktByteNums  period=1  ownership=Locally owned and initialized  arith_type=Unsigned  n_bits=32  bin_pt=0  dbl_ovrd=off  block_type=fromreg
      settings: AttributesFormatString=<< %<shared_memory_name> >>  infoedit="Register block that reads data to a shared memory register.  Delay of one sample period."  block_version=VER_STRING_GOES_HERE
  Slice15 : xbsIndex_r4/Slice  nbits=1  boolean_output=off  mode=Lower Bit Location + Width  bit1=0  base1=MSB of Input  bit0=0  base0=LSB of Input  dbl_ovrd=off  block_type=slice
      settings: infoedit="Extracts a given range of bits from each input sample and presents it at the output.  The output type is ordinarily unsigned with binary point at zero, but can be Boolean when the slice is one bit wide.<P><P>Hardware notes: In hardware this block costs nothing."  block_version=VER_STRING_GOES_HERE
  Slice16 : xbsIndex_r4/Slice  nbits=1  boolean_output=on  mode=Lower Bit Location + Width  bit1=0  base1=MSB of Input  bit0=1  base0=LSB of Input  dbl_ovrd=off  block_type=slice
      settings: infoedit="Extracts a given range of bits from each input sample and presents it at the output.  The output type is ordinarily unsigned with binary point at zero, but can be Boolean when the slice is one bit wide.<P><P>Hardware notes: In hardware this block costs nothing."  block_version=VER_STRING_GOES_HERE
  Slice17 : xbsIndex_r4/Slice  nbits=1  boolean_output=on  mode=Lower Bit Location + Width  bit1=0  base1=MSB of Input  bit0=2  base0=LSB of Input  dbl_ovrd=off  block_type=slice
      settings: infoedit="Extracts a given range of bits from each input sample and presents it at the output.  The output type is ordinarily unsigned with binary point at zero, but can be Boolean when the slice is one bit wide.<P><P>Hardware notes: In hardware this block costs nothing."  block_version=VER_STRING_GOES_HERE
  Slices & Gotos : Subsystem
  ToBool : xbsIndex_r4/Convert  arith_type=Boolean  n_bits=16  bin_pt=14  quantization=Truncate  overflow=Wrap  latency=0  dbl_ovrd=off  pipeline=off  block_type=convert
      settings: block_version=VER_STRING_GOES_HERE
  Tx Slices & Gotos : Subsystem
  TxRx_FFTScaling : xbsIndex_r4/From Register  shared_memory_name='TxRx_FFT_Scaling'  init=TxRx_FFTScaling  period=1  ownership=Locally owned and initialized  arith_type=Unsigned  n_bits=12  bin_pt=0  dbl_ovrd=off  block_type=fromreg
      settings: AttributesFormatString=<< %<shared_memory_name> >>  infoedit="Register block that reads data to a shared memory register.  Delay of one sample period."  block_version=VER_STRING_GOES_HERE
  Tx_PreambleMasking : xbsIndex_r4/From Register  shared_memory_name='Tx_PreambleMasking'  init=0  period=1  ownership=Locally owned and initialized  arith_type=Unsigned  n_bits=32  bin_pt=0  dbl_ovrd=off  block_type=fromreg
      settings: AttributesFormatString=<< %<shared_memory_name> >>  infoedit="Register block that reads data to a shared memory register.  Delay of one sample period."  block_version=VER_STRING_GOES_HERE
  Tx_Reset : xbsIndex_r4/Gateway In  arith_type=Unsigned  n_bits=1  bin_pt=0  quantization="Round  (unbiased: +/- Inf)"  overflow=Saturate  period=4  dbl_ovrd=off  timing_constraint=None  locs_specified=off  LOCs={}  block_type=gatewayin
      settings: infoedit="Gateway in block.  Converts inputs of type Simulink integer, double and fixed point to  Xilinx fixed point type.<P><P>Hardware notes:  In hardware these blocks become top level input ports."  xl_area=[0, 0, 0, 0, 0, 0, 0]  block_version=VER_STRING_GOES_HERE
  Tx_Start_Reset_Control : xbsIndex_r4/From Register  shared_memory_name='Tx_Start_Reset_Control'  init=0  period=1  ownership=Locally owned and initialized  arith_type=Unsigned  n_bits=32  bin_pt=0  dbl_ovrd=off  block_type=fromreg
      settings: AttributesFormatString=<< %<shared_memory_name> >>  infoedit="Register block that reads data to a shared memory register.  Delay of one sample period."  block_version=VER_STRING_GOES_HERE
  Tx_controlBits : xbsIndex_r4/From Register  shared_memory_name='Tx_ControlBits'  init=tx_controlBits  period=1  ownership=Locally owned and initialized  arith_type=Unsigned  n_bits=32  bin_pt=0  dbl_ovrd=off  block_type=fromreg
      settings: AttributesFormatString=<< %<shared_memory_name> >>  infoedit="Register block that reads data to a shared memory register.  Delay of one sample period."  block_version=VER_STRING_GOES_HERE
  tag scope: Rx_FFTScaling=global, Rx_IntPktDet_Thresholds=global, TxReg_PktDoneReset=global, TxReg_PreambleMasking=global, TxReg_TxStart=global, TxReset=global, Tx_FFTScaling=global, regRX_CP_Length=global, regRX_numSubcarriers=global, regRX_sampsPerSymMinusOne=global, regRx_CFOCalc_Dly=global, regRx_PktDet_Delay=global, regRx_PktDet_LongCorr_CountLoad=global, regRx_PktDet_LongCorr_Thresh=global, regRx_PktDone_Reset=global, regRx_headerByteNums=global, regRx_numBaseRateSymbols=global, regRx_numTrainPlusBaseRateSyms=global, regRx_numTrainingSymbols=global, regRx_symbolTimingOffset=global, regTxRx_PktBuffOffsets=global, regTxRx_numHeaderBytes=global, rx_global_reset=global, rx_global_reset_DV2=global
  wires:
  Rx_ControlBits/1 -> Down Sample18/1
  [RegTxRx_CPLength] -> Down Sample8/1
  Constant/1 -> [regRX_numSubcarriers]
  Constant1/1 -> [regRX_sampsPerSymMinusOne]
  AddSub1/1 -> [regRx_numTrainPlusBaseRateSyms]
  Rx_PktDone_Reset/1 -> Down Sample7/1, 4LSB+8/1
  Rx_PktDet_Delay/1 -> 7LSB/1, 5b+16/1, 6LSB+7/1
  RXreset/1 -> Rx_Reset/1
  [regRx_GlobalReset] -> Logical1/1
  Logical1/1 -> [rx_global_reset], Down Sample6/1
  Rx_Reset/1 -> Convert/1
  Convert/1 -> Logical1/2
  Down Sample6/1 -> [rx_global_reset_DV2]
  Down Sample7/1 -> [regRx_PktDone_Reset]
  Down Sample8/1 -> [regRX_CP_Length]
  Down Sample9/1 -> [regRx_PktDet_Delay]
  16LSB1/1 -> [regRx_numTrainingSymbols], AddSub1/1
  16MSB1/1 -> [regRx_numBaseRateSymbols], AddSub1/2
  16MSB/1 -> [regRx_PktDet_LongCorr_Thresh]
  16LSB/1 -> [regRx_PktDet_LongCorr_CountLoad]
  Rx_PktDet_LongCorr_Params/1 -> Down Sample12/1
  Down Sample12/1 -> 16MSB/1, 16LSB/1
  Rx_OFDM_SymbolCounts/1 -> Down Sample11/1
  Down Sample11/1 -> 16MSB1/1, 16LSB1/1
  7LSB/1 -> Down Sample9/1
  6LSB+7/1 -> Down Sample17/1
  Down Sample17/1 -> [regRx_symbolTimingOffset]
  Tx_controlBits/1 -> Down Sample19/1
  Tx_Reset/1 -> Logical/1
  Logical/1 -> ToBool/1
  Slice15/1 -> Logical/2
  ToBool/1 -> [TxReset]
  Constant2/1 -> Tx_Reset/1
  Tx_Start_Reset_Control/1 -> Down Sample20/1
  Slice16/1 -> [TxReg_TxStart]
  Slice17/1 -> [TxReg_PktDoneReset]
  Down Sample20/1 -> Slice15/1, Slice16/1, Slice17/1
  Down Sample23/1 -> [regRx_CFOCalc_Dly]
  5b+16/1 -> Down Sample23/1
  TxRx_FFTScaling/1 -> Down Sample25/1
  Down Sample25/1 -> 6LSB1/1, 6LSB+6/1
  6LSB1/1 -> [Tx_FFTScaling]
  6LSB+6/1 -> [Rx_FFTScaling]
  Constant3/1 -> Down Sample16/1
  Down Sample16/1 -> [Rx_IntPktDet_Thresholds]
  Down Sample18/1 -> Slices & Gotos/1(32b)
  Tx_PreambleMasking/1 -> Down Sample1/1
  Down Sample1/1 -> [TxReg_PreambleMasking]
  Rx_pktByteNums/1 -> Down Sample2/1
  Down Sample2/1 -> 24MSB/1, "8LSB "/1
  24MSB/1 -> [regRx_headerByteNums]
  Down Sample19/1 -> Tx Slices & Gotos/1(32b)
  4LSB+8/1 -> [regTxRx_PktBuffOffsets]
  "8LSB "/1 -> [regTxRx_numHeaderBytes]

== system ofdm_txrx_alamouti/Workspace Transmitter ==
  From Workspace : FromWorkspace  VariableName=rxAntI  SampleTime=4  Interpolate=off  OutputAfterFinalValue=Cyclic repetition
  From Workspace1 : FromWorkspace  VariableName=rxAntQ  SampleTime=4  Interpolate=off  OutputAfterFinalValue=Cyclic repetition
  RXreset : Constant  Value=0  SampleTime=4
  A_I : Outport
  A_Q : Outport  Port=2
  B_I : Outport  Port=3
  B_Q : Outport  Port=4
  wires:
  From Workspace/1 -> A_I/1
  From Workspace1/1 -> A_Q/1
  RXreset/1 -> B_I/1, B_Q/1

== system ofdm_txrx_alamouti/"Channel"/Freq Offset ==
  note: Optional additive carrier
      | Models effects of DC-offsets at Tx/Rx
      | Set gain=0.15 for realistic offset
  In1 : Inport
  In2 : Inport  Port=2
  Gain : Gain  Orientation=up  Gain=0
  Product4 : Product  RndMeth=Zero
  Product5 : Product  RndMeth=Zero
  Sine Wave : dspsrcs4/Sine Wave  Amplitude=1  Frequency=freqOffset/4  Phase=0  SampleMode=Discrete  OutComplex=Complex  CompMethod=Trigonometric fcn  TableSize=Speed  SampleTime=1  SamplesPerFrame=1  dataType=double  wordLen=16  udDataType=sfix(16)  fracBitsMode=Best precision  numFracBits=15  ResetState=Restart at time zero
  Sum : Sum  Inputs=|++
  Sum1 : Sum  Inputs=|++
  Out1 : Outport
  Out2 : Outport  Port=2
  tag scope: freqOffset=global
  wires:
  Sine Wave/1 -> Product4/2, Product5/2, [freqOffset], Gain/1
  In1/1 -> Product4/1
  Product4/1 -> Sum/1
  In2/1 -> Product5/1
  Product5/1 -> Sum1/1
  Sum/1 -> Out1/1
  Sum1/1 -> Out2/1
  Gain/1 -> Sum1/2, Sum/2

== system ofdm_txrx_alamouti/"Channel"/MIMO "Channel" ==
  note: Channel matrix is 2x2 of complex scalars.
      | This block implements Y=HX where
      | X = [TxA;TxB]
      | H = [h_AA h_BA; h_AB h_BB]
      | Y = [RxA; RxB]
      | RxA = TxA*h_AA + TxB*h_BA
      | RxB = TxB*h_BB + TxA*h_AB
      | where
      | TxA is signal from Tx antenna A
      | TxB is signal from Tx antenna B
      | RxA is signal at Rx antenna A
      | RxB is signal at Rx antenna B
      | h_AA is channel for TxA -> RxA
      | h_AB is channel for TxA -> RxB
      | h_BA is channel for TxB -> RxA
      | h_BB is channel for TxB -> RxB
  note: h_AA
  note: h_BA
  note: h_AB
  note: h_BB
  TxA : Inport
  TxB : Inport  Port=2
  Add : Sum  OutScaling=2^-10
  Add1 : Sum  OutScaling=2^-10
  Constant : Constant  Value=h(1,1)
  Constant1 : Constant  Value=h(1,2)
  Constant2 : Constant  Value=h(2,1)
  Constant3 : Constant  Value=h(2,2)
  Display{,1..3} (4 alike) : Display
  Product{,1..3} (4 alike) : Product  RndMeth=Zero
  RxA : Outport
  RxB : Outport  Port=2
  wires:
  Constant/1 -> Product/2, Display/1
  Product/1 -> Add/1
  TxA/1 -> Product2/1, Product/1
  TxB/1 -> Product1/2, Product3/2
  Constant1/1 -> Product1/1, Display1/1
  Product1/1 -> Add/2
  Constant2/1 -> Product2/2, Display2/1
  Product2/1 -> Add1/1
  Constant3/1 -> Product3/1, Display3/1
  Product3/1 -> Add1/2
  Add/1 -> RxA/1
  Add1/1 -> RxB/1
